simulink model "fft_test_BG"  (Simulink 7.4)
settings: Solver=ode45  StartTime=0.0  StopTime=5000  RelTol=1e-3
libraries: xbsIndex_r4 x4277, casper_library_misc x172, xbsReplacements_r4 x47, casper_library_delays x21, casper_library_reorder x4, casper_library_ffts_twiddle x2, simulink x2, xps_library x1
(part 37 of 37)

== system fft_test_BG/fft_BG_xblock/fft_biplex2/biplex_core/fft_stage_8/butterfly_direct/twiddle/c_to_ri_a ==
  c : Inport
  force_im : xbsIndex_r4/Reinterpret  force_arith_type=on  arith_type="Signed  (2's comp)"  force_bin_pt=on  bin_pt=15  block_type=reinterpret
      settings: infoedit="Changes signal type without altering the binary representation.   You can changed the signal between signed and unsigned, and relocate the binary point.<br><br>Hardware notes: In hardware this block costs nothing.<br><br>Example:  Suppose the input is 6 bits wide, signed, with 2 fractional bits, and the output is forced to unsigned with 0 fractional bits.  Then an input of -2.0 (1110.00 in binary 2's complement) becomes an output of 56 (111000 in binary)."
  force_re : xbsIndex_r4/Reinterpret  force_arith_type=on  arith_type="Signed  (2's comp)"  force_bin_pt=on  bin_pt=15  block_type=reinterpret
      settings: infoedit="Changes signal type without altering the binary representation.   You can changed the signal between signed and unsigned, and relocate the binary point.<br><br>Hardware notes: In hardware this block costs nothing.<br><br>Example:  Suppose the input is 6 bits wide, signed, with 2 fractional bits, and the output is forced to unsigned with 0 fractional bits.  Then an input of -2.0 (1110.00 in binary 2's complement) becomes an output of 56 (111000 in binary)."
  slice_im : xbsIndex_r4/Slice  nbits=16  boolean_output=off  mode=Lower Bit Location + Width  bit1=0  base1=MSB of Input  bit0=0  base0=LSB of Input  dbl_ovrd=off  block_type=slice
  slice_re : xbsIndex_r4/Slice  nbits=16  boolean_output=off  mode=Upper Bit Location + Width  bit1=0  base1=MSB of Input  bit0=0  base0=LSB of Input  dbl_ovrd=off  block_type=slice
  re : Outport
  im : Outport  Port=2
  wires:
  c/1 -> slice_re/1, slice_im/1
  slice_re/1 -> force_re/1
  slice_im/1 -> force_im/1
  force_re/1 -> re/1
  force_im/1 -> im/1

== system fft_test_BG/fft_BG_xblock/fft_biplex2/biplex_core/fft_stage_8/butterfly_direct/twiddle/c_to_ri_b ==
  c : Inport
  force_im : xbsIndex_r4/Reinterpret  force_arith_type=on  arith_type="Signed  (2's comp)"  force_bin_pt=on  bin_pt=15  block_type=reinterpret
      settings: infoedit="Changes signal type without altering the binary representation.   You can changed the signal between signed and unsigned, and relocate the binary point.<br><br>Hardware notes: In hardware this block costs nothing.<br><br>Example:  Suppose the input is 6 bits wide, signed, with 2 fractional bits, and the output is forced to unsigned with 0 fractional bits.  Then an input of -2.0 (1110.00 in binary 2's complement) becomes an output of 56 (111000 in binary)."
  force_re : xbsIndex_r4/Reinterpret  force_arith_type=on  arith_type="Signed  (2's comp)"  force_bin_pt=on  bin_pt=15  block_type=reinterpret
      settings: infoedit="Changes signal type without altering the binary representation.   You can changed the signal between signed and unsigned, and relocate the binary point.<br><br>Hardware notes: In hardware this block costs nothing.<br><br>Example:  Suppose the input is 6 bits wide, signed, with 2 fractional bits, and the output is forced to unsigned with 0 fractional bits.  Then an input of -2.0 (1110.00 in binary 2's complement) becomes an output of 56 (111000 in binary)."
  slice_im : xbsIndex_r4/Slice  nbits=16  boolean_output=off  mode=Lower Bit Location + Width  bit1=0  base1=MSB of Input  bit0=0  base0=LSB of Input  dbl_ovrd=off  block_type=slice
  slice_re : xbsIndex_r4/Slice  nbits=16  boolean_output=off  mode=Upper Bit Location + Width  bit1=0  base1=MSB of Input  bit0=0  base0=LSB of Input  dbl_ovrd=off  block_type=slice
  re : Outport
  im : Outport  Port=2
  wires:
  c/1 -> slice_re/1, slice_im/1
  slice_re/1 -> force_re/1
  slice_im/1 -> force_im/1
  force_re/1 -> re/1
  force_im/1 -> im/1

== system fft_test_BG/fft_BG_xblock/fft_biplex2/biplex_core/fft_stage_8/butterfly_direct/twiddle/c_to_ri_w ==
  c : Inport
  force_im : xbsIndex_r4/Reinterpret  force_arith_type=on  arith_type="Signed  (2's comp)"  force_bin_pt=on  bin_pt=14  block_type=reinterpret
      settings: infoedit="Changes signal type without altering the binary representation.   You can changed the signal between signed and unsigned, and relocate the binary point.<br><br>Hardware notes: In hardware this block costs nothing.<br><br>Example:  Suppose the input is 6 bits wide, signed, with 2 fractional bits, and the output is forced to unsigned with 0 fractional bits.  Then an input of -2.0 (1110.00 in binary 2's complement) becomes an output of 56 (111000 in binary)."
  force_re : xbsIndex_r4/Reinterpret  force_arith_type=on  arith_type="Signed  (2's comp)"  force_bin_pt=on  bin_pt=14  block_type=reinterpret
      settings: infoedit="Changes signal type without altering the binary representation.   You can changed the signal between signed and unsigned, and relocate the binary point.<br><br>Hardware notes: In hardware this block costs nothing.<br><br>Example:  Suppose the input is 6 bits wide, signed, with 2 fractional bits, and the output is forced to unsigned with 0 fractional bits.  Then an input of -2.0 (1110.00 in binary 2's complement) becomes an output of 56 (111000 in binary)."
  slice_im : xbsIndex_r4/Slice  nbits=16  boolean_output=off  mode=Lower Bit Location + Width  bit1=0  base1=MSB of Input  bit0=0  base0=LSB of Input  dbl_ovrd=off  block_type=slice
  slice_re : xbsIndex_r4/Slice  nbits=16  boolean_output=off  mode=Upper Bit Location + Width  bit1=0  base1=MSB of Input  bit0=0  base0=LSB of Input  dbl_ovrd=off  block_type=slice
  re : Outport
  im : Outport  Port=2
  wires:
  c/1 -> slice_re/1, slice_im/1
  slice_re/1 -> force_re/1
  slice_im/1 -> force_im/1
  force_re/1 -> re/1
  force_im/1 -> im/1

== system fft_test_BG/fft_BG_xblock/fft_biplex2/biplex_core/fft_stage_8/butterfly_direct/twiddle/coeff_gen ==
  rst : Inport
  Counter : xbsIndex_r4/Counter  cnt_type=Free Running  cnt_to=Inf  operation=Up  start_count=0  cnt_by_val=1  arith_type=Unsigned  n_bits=7  bin_pt=0  load_pin=off  rst=on  en=off  explicit_period=on  period=1  dbl_ovrd=off  use_behavioral_HDL=off  implementation=Fabric  block_type=counter
      settings: block_version=11.4
  ROM : xbsIndex_r4/ROM  depth=128  initVector=[1 0 0.70709228515625 -0.70709228515625 0.92388916015625 -0.3826904296875 0.3826904296875 -0.92388916015625 0.98077392578125 -0.195068359375 0.5555419921875 -0.83148193359375 0.83148193359375 -0.5555419921875 0.195068359375 -0.98077392578125 0.99517822265625 -0.0980224609375 0.6343994140625 -0.77301025390625 0.88189697265625 -0.47137451171875 0.290283203125 -0.95697021484375 0.95697021484375 -0.290283203125 0.47137451171875 -0.88189697265625 0.77301025390625 -0.6343994140625 0.0980224609375 -0.99517822265625 0.998779296875 -0.049072265625 0.67156982421875 -0.740966796875 0.90399169921875 -0.42755126953125 0.3369140625 -0.9415283203125 0.97003173828125 -0.24298095703125 0.51409912109375 -0.85772705078125 0.80322265625 -0.595703125 0.146728515625 -0.98919677734375 0.98919677734375 -0.146728515625 0.595703125 -0.80322265625 0.85772705078125 -0.51409912109375 0.24298095703125 -0.97003173828125 0.9415283203125 -0.3369140625 0.42755126953125 -0.90399169921875 0.740966796875 -0.67156982421875 0.049072265625 -0.998779296875 0.99969482421875 -0.0245361328125 0.68951416015625 -0.7242431640625 0.9141845703125 -0.40521240234375 0.35992431640625 -0.9329833984375 0.9757080078125 -0.2191162109375 0.53497314453125 -0.8448486328125 0.81756591796875 -0.5758056640625 0.17095947265625 -0.98529052734375 0.99249267578125 -0.1224365234375 0.615234375 -0.788330078125 0.8701171875 -0.492919921875 0.2667236328125 -0.96380615234375 0.94952392578125 -0.31365966796875 0.4495849609375 -0.89324951171875 0.7572021484375 -0.6531982421875 0.07354736328125 -0.997314453125 0.997314453125 -0.07354736328125 0.6531982421875 -0.7572021484375 0.89324951171875 -0.4495849609375 0.31365966796875 -0.94952392578125 0.96380615234375 -0.2667236328125 0.492919921875 -0.8701171875 0.788330078125 -0.615234375 0.1224365234375 -0.99249267578125 0.98529052734375 -0.17095947265625 0.5758056640625 -0.81756591796875 0.8448486328125 -0.53497314453125 0.2191162109375 -0.9757080078125 0.9329833984375 -0.35992431640625 0.40521240234375 -0.9141845703125 0.7242431640625 -0.68951416015625 0.0245361328125 -0.99969482421875]  distributed_mem=Block RAM  rst=off  init_reg=0  en=off  latency=2  arith_type="Signed  (2's comp)"  n_bits=16  bin_pt=14  dbl_ovrd=off  optimize=Area  use_rpm=on  block_type=sprom
      settings: block_version=11.4
  ROM1 : xbsIndex_r4/ROM  depth=128  initVector=[0 -1 -0.70709228515625 -0.70709228515625 -0.3826904296875 -0.92388916015625 -0.92388916015625 -0.3826904296875 -0.195068359375 -0.98077392578125 -0.83148193359375 -0.5555419921875 -0.5555419921875 -0.83148193359375 -0.98077392578125 -0.195068359375 -0.0980224609375 -0.99517822265625 -0.77301025390625 -0.6343994140625 -0.47137451171875 -0.88189697265625 -0.95697021484375 -0.290283203125 -0.290283203125 -0.95697021484375 -0.88189697265625 -0.47137451171875 -0.6343994140625 -0.77301025390625 -0.99517822265625 -0.0980224609375 -0.049072265625 -0.998779296875 -0.740966796875 -0.67156982421875 -0.42755126953125 -0.90399169921875 -0.9415283203125 -0.3369140625 -0.24298095703125 -0.97003173828125 -0.85772705078125 -0.51409912109375 -0.595703125 -0.80322265625 -0.98919677734375 -0.146728515625 -0.146728515625 -0.98919677734375 -0.80322265625 -0.595703125 -0.51409912109375 -0.85772705078125 -0.97003173828125 -0.24298095703125 -0.3369140625 -0.9415283203125 -0.90399169921875 -0.42755126953125 -0.67156982421875 -0.740966796875 -0.998779296875 -0.049072265625 -0.0245361328125 -0.99969482421875 -0.7242431640625 -0.68951416015625 -0.40521240234375 -0.9141845703125 -0.9329833984375 -0.35992431640625 -0.2191162109375 -0.9757080078125 -0.8448486328125 -0.53497314453125 -0.5758056640625 -0.81756591796875 -0.98529052734375 -0.17095947265625 -0.1224365234375 -0.99249267578125 -0.788330078125 -0.615234375 -0.492919921875 -0.8701171875 -0.96380615234375 -0.2667236328125 -0.31365966796875 -0.94952392578125 -0.89324951171875 -0.4495849609375 -0.6531982421875 -0.7572021484375 -0.997314453125 -0.07354736328125 -0.07354736328125 -0.997314453125 -0.7572021484375 -0.6531982421875 -0.4495849609375 -0.89324951171875 -0.94952392578125 -0.31365966796875 -0.2667236328125 -0.96380615234375 -0.8701171875 -0.492919921875 -0.615234375 -0.788330078125 -0.99249267578125 -0.1224365234375 -0.17095947265625 -0.98529052734375 -0.81756591796875 -0.5758056640625 -0.53497314453125 -0.8448486328125 -0.9757080078125 -0.2191162109375 -0.35992431640625 -0.9329833984375 -0.9141845703125 -0.40521240234375 -0.68951416015625 -0.7242431640625 -0.99969482421875 -0.0245361328125]  distributed_mem=Block RAM  rst=off  init_reg=0  en=off  latency=2  arith_type="Signed  (2's comp)"  n_bits=16  bin_pt=14  dbl_ovrd=off  optimize=Area  use_rpm=on  block_type=sprom
      settings: block_version=11.4
  Slice : xbsIndex_r4/Slice  nbits=7  boolean_output=off  mode=Upper Bit Location + Width  bit1=0  base1=MSB of Input  bit0=0  base0=LSB of Input  dbl_ovrd=off  block_type=slice
  ri_to_c : casper_library_misc/ri_to_c  SystemSampleTime=-1
  w : Outport
  wires:
  Slice/1 -> ROM1/1, ROM/1
  ri_to_c/1 -> w/1
  ROM1/1 -> ri_to_c/2
  ROM/1 -> ri_to_c/1
  Counter/1 -> Slice/1
  rst/1 -> Counter/1

== system fft_test_BG/fft_BG_xblock/fft_biplex2/biplex_core/fft_stage_8/butterfly_direct/twiddle/mults ==
  a1 : Inport
  b1 : Inport  Port=2
  a2 : Inport  Port=3
  b2 : Inport  Port=4
  a3 : Inport  Port=5
  b3 : Inport  Port=6
  a4 : Inport  Port=7
  b4 : Inport  Port=8
  mult_{1..4} (4 alike) : xbsIndex_r4/Mult  precision=Full  arith_type="Signed  (2's comp)"  n_bits=16  bin_pt=14  quantization="Round  (unbiased: +/- Inf)"  overflow=Saturate  en=off  latency=2  dbl_ovrd=off  use_behavioral_HDL=off  opt=Speed  use_embedded=on  optimum_pipeline=off  pipeline=on  use_rpm=on  placement_style=Triangular  block_type=mult
      settings: block_version=11.4
  c1 : Outport
  c2 : Outport  Port=2
  c3 : Outport  Port=3
  c4 : Outport  Port=4
  wires:
  mult_4/1 -> c4/1
  b4/1 -> mult_4/2
  a4/1 -> mult_4/1
  mult_3/1 -> c3/1
  b3/1 -> mult_3/2
  a3/1 -> mult_3/1
  mult_2/1 -> c2/1
  b2/1 -> mult_2/2
  a2/1 -> mult_2/1
  mult_1/1 -> c1/1
  b1/1 -> mult_1/2
  a1/1 -> mult_1/1
